simulink library "inp"  (Simulink 8.2) (continued)
== system inp/INP/InputSignals/SignalProcess/Proc_CameraSignals == (continued)
  [VINP_Obstacle6_BUS_InHAL] -> Bus Selector55/1
  [VINP_Obstacle7_BUS_InHAL] -> Bus Selector56/1
  [VINP_Obstacle8_BUS_InHAL] -> Bus Selector57/1
  [VINP_Obstacle9_BUS_InHAL] -> Bus Selector58/1
  [VINP_Obstacle10_BUS_InHAL] -> Bus Selector59/1
  Bus Selector59/1 -> Mux5/10  "<VINP_CamObs10PosYValid_flg_InHAL>"
  Mux5/1 -> VINP_CamObsPosYValid10_flg_InProc/1  "VINP_CamObsPosYValid10_flg_InProc"
  Bus Selector60/1 -> Mux6/1  "<VINP_CamObs1VelX_mps_InHAL>"
  Bus Selector61/1 -> Mux6/2  "<VINP_CamObs2VelX_mps_InHAL>"
  Bus Selector62/1 -> Mux6/3  "<VINP_CamObs3VelX_mps_InHAL>"
  Bus Selector63/1 -> Mux6/4  "<VINP_CamObs4VelX_mps_InHAL>"
  Bus Selector64/1 -> Mux6/5  "<VINP_CamObs5VelX_mps_InHAL>"
  Bus Selector65/1 -> Mux6/6  "<VINP_CamObs6VelX_mps_InHAL>"
  Bus Selector66/1 -> Mux6/7  "<VINP_CamObs7VelX_mps_InHAL>"
  Bus Selector67/1 -> Mux6/8  "<VINP_CamObs8VelX_mps_InHAL>"
  Bus Selector68/1 -> Mux6/9  "<VINP_CamObs9VelX_mps_InHAL>"
  [VINP_Obstacle1_BUS_InHAL] -> Bus Selector60/1
  [VINP_Obstacle2_BUS_InHAL] -> Bus Selector61/1
  [VINP_Obstacle3_BUS_InHAL] -> Bus Selector62/1
  [VINP_Obstacle4_BUS_InHAL] -> Bus Selector63/1
  [VINP_Obstacle5_BUS_InHAL] -> Bus Selector64/1
  [VINP_Obstacle6_BUS_InHAL] -> Bus Selector65/1
  [VINP_Obstacle7_BUS_InHAL] -> Bus Selector66/1
  [VINP_Obstacle8_BUS_InHAL] -> Bus Selector67/1
  [VINP_Obstacle9_BUS_InHAL] -> Bus Selector68/1
  [VINP_Obstacle10_BUS_InHAL] -> Bus Selector69/1
  Bus Selector69/1 -> Mux6/10  "<VINP_CamObs10VelX_mps_InHAL>"
  Mux6/1 -> VINP_CamObsVelX10_mps_InProc/1  "VINP_CamObsVelX10_mps_InProc"
  Bus Selector70/1 -> Mux7/1  "<VINP_CamObs1VelXValid_flg_InHAL>"
  Bus Selector71/1 -> Mux7/2  "<VINP_CamObs2VelXValid_flg_InHAL>"
  Bus Selector72/1 -> Mux7/3  "<VINP_CamObs3VelXValid_flg_InHAL>"
  Bus Selector73/1 -> Mux7/4  "<VINP_CamObs4VelXValid_flg_InHAL>"
  Bus Selector74/1 -> Mux7/5  "<VINP_CamObs5VelXValid_flg_InHAL>"
  Bus Selector75/1 -> Mux7/6  "<VINP_CamObs6VelXValid_flg_InHAL>"
  Bus Selector76/1 -> Mux7/7  "<VINP_CamObs7VelXValid_flg_InHAL>"
  Bus Selector77/1 -> Mux7/8  "<VINP_CamObs8VelXValid_flg_InHAL>"
  Bus Selector78/1 -> Mux7/9  "<VINP_CamObs9VelXValid_flg_InHAL>"
  [VINP_Obstacle1_BUS_InHAL] -> Bus Selector70/1
  [VINP_Obstacle2_BUS_InHAL] -> Bus Selector71/1
  [VINP_Obstacle3_BUS_InHAL] -> Bus Selector72/1
  [VINP_Obstacle4_BUS_InHAL] -> Bus Selector73/1
  [VINP_Obstacle5_BUS_InHAL] -> Bus Selector74/1
  [VINP_Obstacle6_BUS_InHAL] -> Bus Selector75/1
  [VINP_Obstacle7_BUS_InHAL] -> Bus Selector76/1
  [VINP_Obstacle8_BUS_InHAL] -> Bus Selector77/1
  [VINP_Obstacle9_BUS_InHAL] -> Bus Selector78/1
  [VINP_Obstacle10_BUS_InHAL] -> Bus Selector79/1
  Bus Selector79/1 -> Mux7/10  "<VINP_CamObs10VelXValid_flg_InHAL>"
  Mux7/1 -> VINP_CamObsVelXValid10_flg_InProc/1  "VINP_CamObsVelXValid10_flg_InProc"
  Bus Selector80/1 -> Mux8/1  "<VINP_CamObs1Type_enum_InHAL>"
  Bus Selector81/1 -> Mux8/2  "<VINP_CamObs2Type_enum_InHAL>"
  Bus Selector82/1 -> Mux8/3  "<VINP_CamObs3Type_enum_InHAL>"
  Bus Selector83/1 -> Mux8/4  "<VINP_CamObs4Type_enum_InHAL>"
  Bus Selector84/1 -> Mux8/5  "<VINP_CamObs5Type_enum_InHAL>"
  Bus Selector85/1 -> Mux8/6  "<VINP_CamObs6Type_enum_InHAL>"
  Bus Selector86/1 -> Mux8/7  "<VINP_CamObs7Type_enum_InHAL>"
  Bus Selector87/1 -> Mux8/8  "<VINP_CamObs8Type_enum_InHAL>"
  Bus Selector88/1 -> Mux8/9  "<VINP_CamObs9Type_enum_InHAL>"
  [VINP_Obstacle1_BUS_InHAL] -> Bus Selector80/1
  [VINP_Obstacle2_BUS_InHAL] -> Bus Selector81/1
  [VINP_Obstacle3_BUS_InHAL] -> Bus Selector82/1
  [VINP_Obstacle4_BUS_InHAL] -> Bus Selector83/1
  [VINP_Obstacle5_BUS_InHAL] -> Bus Selector84/1
  [VINP_Obstacle6_BUS_InHAL] -> Bus Selector85/1
  [VINP_Obstacle7_BUS_InHAL] -> Bus Selector86/1
  [VINP_Obstacle8_BUS_InHAL] -> Bus Selector87/1
  [VINP_Obstacle9_BUS_InHAL] -> Bus Selector88/1
  [VINP_Obstacle10_BUS_InHAL] -> Bus Selector89/1
  Bus Selector89/1 -> Mux8/10  "<VINP_CamObs10Type_enum_InHAL>"
  Mux8/1 -> VINP_CamObsType10_enum_InProc/1  "VINP_CamObsType10_enum_InProc"
  Bus Selector90/1 -> Mux9/1  "<VINP_CamObs1TypeValid_flg_InHAL>"
  Bus Selector91/1 -> Mux9/2  "<VINP_CamObs2TypeValid_flg_InHAL>"
  Bus Selector92/1 -> Mux9/3  "<VINP_CamObs3TypeValid_flg_InHAL>"
  Bus Selector93/1 -> Mux9/4  "<VINP_CamObs4TypeValid_flg_InHAL>"
  Bus Selector94/1 -> Mux9/5  "<VINP_CamObs5TypeValid_flg_InHAL>"
  Bus Selector95/1 -> Mux9/6  "<VINP_CamObs6TypeValid_flg_InHAL>"
  Bus Selector96/1 -> Mux9/7  "<VINP_CamObs7TypeValid_flg_InHAL>"
  Bus Selector97/1 -> Mux9/8  "<VINP_CamObs8TypeValid_flg_InHAL>"
  Bus Selector98/1 -> Mux9/9  "<VINP_CamObs9TypeValid_flg_InHAL>"
  [VINP_Obstacle1_BUS_InHAL] -> Bus Selector90/1
  [VINP_Obstacle2_BUS_InHAL] -> Bus Selector91/1
  [VINP_Obstacle3_BUS_InHAL] -> Bus Selector92/1
  [VINP_Obstacle4_BUS_InHAL] -> Bus Selector93/1
  [VINP_Obstacle5_BUS_InHAL] -> Bus Selector94/1
  [VINP_Obstacle6_BUS_InHAL] -> Bus Selector95/1
  [VINP_Obstacle7_BUS_InHAL] -> Bus Selector96/1
  [VINP_Obstacle8_BUS_InHAL] -> Bus Selector97/1
  [VINP_Obstacle9_BUS_InHAL] -> Bus Selector98/1
  [VINP_Obstacle10_BUS_InHAL] -> Bus Selector99/1
  Bus Selector99/1 -> Mux9/10  "<VINP_CamObs10TypeValid_flg_InHAL>"
  Mux9/1 -> VINP_CamObsTypeValid10_flg_InProc/1  "VINP_CamObsTypeValid10_flg_InProc"
  Bus Selector100/1 -> Mux10/1  "<VINP_CamObs1Status_enum_InHAL>"
  Bus Selector101/1 -> Mux10/2  "<VINP_CamObs2Status_enum_InHAL>"
  Bus Selector102/1 -> Mux10/3  "<VINP_CamObs3Status_enum_InHAL>"
  Bus Selector103/1 -> Mux10/4  "<VINP_CamObs4Status_enum_InHAL>"
  Bus Selector104/1 -> Mux10/5  "<VINP_CamObs5Status_enum_InHAL>"
  Bus Selector105/1 -> Mux10/6  "<VINP_CamObs6Status_enum_InHAL>"
  Bus Selector106/1 -> Mux10/7  "<VINP_CamObs7Status_enum_InHAL>"
  Bus Selector107/1 -> Mux10/8  "<VINP_CamObs8Status_enum_InHAL>"
  Bus Selector108/1 -> Mux10/9  "<VINP_CamObs9Status_enum_InHAL>"
  [VINP_Obstacle1_BUS_InHAL] -> Bus Selector100/1
  [VINP_Obstacle2_BUS_InHAL] -> Bus Selector101/1
  [VINP_Obstacle3_BUS_InHAL] -> Bus Selector102/1
  [VINP_Obstacle4_BUS_InHAL] -> Bus Selector103/1
  [VINP_Obstacle5_BUS_InHAL] -> Bus Selector104/1
  [VINP_Obstacle6_BUS_InHAL] -> Bus Selector105/1
  [VINP_Obstacle7_BUS_InHAL] -> Bus Selector106/1
  [VINP_Obstacle8_BUS_InHAL] -> Bus Selector107/1
  [VINP_Obstacle9_BUS_InHAL] -> Bus Selector108/1
  [VINP_Obstacle10_BUS_InHAL] -> Bus Selector109/1
  Bus Selector109/1 -> Mux10/10  "<VINP_CamObs10Status_enum_InHAL>"
  Mux10/1 -> VINP_CamObsStatus10_enum_InProc/1  "VINP_CamObsStatus10_enum_InProc"
  Bus Selector110/1 -> Mux11/1  "<VINP_CamObs1StatusValid_flg_InHAL>"
  Bus Selector111/1 -> Mux11/2  "<VINP_CamObs2StatusValid_flg_InHAL>"
  Bus Selector112/1 -> Mux11/3  "<VINP_CamObs3StatusValid_flg_InHAL>"
  Bus Selector113/1 -> Mux11/4  "<VINP_CamObs4StatusValid_flg_InHAL>"
  Bus Selector114/1 -> Mux11/5  "<VINP_CamObs5StatusValid_flg_InHAL>"
  Bus Selector115/1 -> Mux11/6  "<VINP_CamObs6StatusValid_flg_InHAL>"
  Bus Selector116/1 -> Mux11/7  "<VINP_CamObs7StatusValid_flg_InHAL>"
  Bus Selector117/1 -> Mux11/8  "<VINP_CamObs8StatusValid_flg_InHAL>"
  Bus Selector118/1 -> Mux11/9  "<VINP_CamObs9StatusValid_flg_InHAL>"
  [VINP_Obstacle1_BUS_InHAL] -> Bus Selector110/1
  [VINP_Obstacle2_BUS_InHAL] -> Bus Selector111/1
  [VINP_Obstacle3_BUS_InHAL] -> Bus Selector112/1
  [VINP_Obstacle4_BUS_InHAL] -> Bus Selector113/1
  [VINP_Obstacle5_BUS_InHAL] -> Bus Selector114/1
  [VINP_Obstacle6_BUS_InHAL] -> Bus Selector115/1
  [VINP_Obstacle7_BUS_InHAL] -> Bus Selector116/1
  [VINP_Obstacle8_BUS_InHAL] -> Bus Selector117/1
  [VINP_Obstacle9_BUS_InHAL] -> Bus Selector118/1
  [VINP_Obstacle10_BUS_InHAL] -> Bus Selector119/1
  Bus Selector119/1 -> Mux11/10  "<VINP_CamObs10StatusValid_flg_InHAL>"
  Mux11/1 -> VINP_CamObsStatusValid10_flg_InProc/1  "VINP_CamObsStatusValid10_flg_InProc"
  Bus Selector120/1 -> Mux12/1  "<VINP_CamObs1Valid_enum_InHAL>"
  Bus Selector121/1 -> Mux12/2  "<VINP_CamObs2Valid_enum_InHAL>"
  Bus Selector122/1 -> Mux12/3  "<VINP_CamObs3Valid_enum_InHAL>"
  Bus Selector123/1 -> Mux12/4  "<VINP_CamObs4Valid_enum_InHAL>"
  Bus Selector124/1 -> Mux12/5  "<VINP_CamObs5Valid_enum_InHAL>"
  Bus Selector125/1 -> Mux12/6  "<VINP_CamObs6Valid_enum_InHAL>"
  Bus Selector126/1 -> Mux12/7  "<VINP_CamObs7Valid_enum_InHAL>"
  Bus Selector127/1 -> Mux12/8  "<VINP_CamObs8Valid_enum_InHAL>"
  Bus Selector128/1 -> Mux12/9  "<VINP_CamObs9Valid_enum_InHAL>"
  [VINP_Obstacle1_BUS_InHAL] -> Bus Selector120/1
  [VINP_Obstacle2_BUS_InHAL] -> Bus Selector121/1
  [VINP_Obstacle3_BUS_InHAL] -> Bus Selector122/1
  [VINP_Obstacle4_BUS_InHAL] -> Bus Selector123/1
  [VINP_Obstacle5_BUS_InHAL] -> Bus Selector124/1
  [VINP_Obstacle6_BUS_InHAL] -> Bus Selector125/1
  [VINP_Obstacle7_BUS_InHAL] -> Bus Selector126/1
  [VINP_Obstacle8_BUS_InHAL] -> Bus Selector127/1
  [VINP_Obstacle9_BUS_InHAL] -> Bus Selector128/1
  [VINP_Obstacle10_BUS_InHAL] -> Bus Selector129/1
  Bus Selector129/1 -> Mux12/10  "<VINP_CamObs10Valid_enum_InHAL>"
  Mux12/1 -> VINP_CamObsValid10_enum_InProc/1  "VINP_CamObsValid10_enum_InProc"
  Bus Selector130/1 -> Mux13/1  "<VINP_CamObs1ValidValid_flg_InHAL>"
  Bus Selector131/1 -> Mux13/2  "<VINP_CamObs2ValidValid_flg_InHAL>"
  Bus Selector132/1 -> Mux13/3  "<VINP_CamObs3ValidValid_flg_InHAL>"
  Bus Selector133/1 -> Mux13/4  "<VINP_CamObs4ValidValid_flg_InHAL>"
  Bus Selector134/1 -> Mux13/5  "<VINP_CamObs5ValidValid_flg_InHAL>"
  Bus Selector135/1 -> Mux13/6  "<VINP_CamObs6ValidValid_flg_InHAL>"
  Bus Selector136/1 -> Mux13/7  "<VINP_CamObs7ValidValid_flg_InHAL>"
  Bus Selector137/1 -> Mux13/8  "<VINP_CamObs8ValidValid_flg_InHAL>"
  Bus Selector138/1 -> Mux13/9  "<VINP_CamObs9ValidValid_flg_InHAL>"
  [VINP_Obstacle1_BUS_InHAL] -> Bus Selector130/1
  [VINP_Obstacle2_BUS_InHAL] -> Bus Selector131/1
  [VINP_Obstacle3_BUS_InHAL] -> Bus Selector132/1
  [VINP_Obstacle4_BUS_InHAL] -> Bus Selector133/1
  [VINP_Obstacle5_BUS_InHAL] -> Bus Selector134/1
  [VINP_Obstacle6_BUS_InHAL] -> Bus Selector135/1
  [VINP_Obstacle7_BUS_InHAL] -> Bus Selector136/1
  [VINP_Obstacle8_BUS_InHAL] -> Bus Selector137/1
  [VINP_Obstacle9_BUS_InHAL] -> Bus Selector138/1
  [VINP_Obstacle10_BUS_InHAL] -> Bus Selector139/1
  Bus Selector139/1 -> Mux13/10  "<VINP_CamObs10ValidValid_flg_InHAL>"
  Mux13/1 -> VINP_CamObsValidValid10_flg_InProc/1  "VINP_CamObsValidValid10_flg_InProc"
  Bus Selector140/1 -> Mux14/1  "<VINP_CamObs1MoveInO_enum_InHAL>"
  Bus Selector141/1 -> Mux14/2  "<VINP_CamObs2MoveInO_enum_InHAL>"
  Bus Selector142/1 -> Mux14/3  "<VINP_CamObs3MoveInO_enum_InHAL>"
  Bus Selector143/1 -> Mux14/4  "<VINP_CamObs4MoveInO_enum_InHAL>"
  Bus Selector144/1 -> Mux14/5  "<VINP_CamObs5MoveInO_enum_InHAL>"
  Bus Selector145/1 -> Mux14/6  "<VINP_CamObs6MoveInO_enum_InHAL>"
  Bus Selector146/1 -> Mux14/7  "<VINP_CamObs7MoveInO_enum_InHAL>"
  Bus Selector147/1 -> Mux14/8  "<VINP_CamObs8MoveInO_enum_InHAL>"
  Bus Selector148/1 -> Mux14/9  "<VINP_CamObs9MoveInO_enum_InHAL>"
  [VINP_Obstacle1_BUS_InHAL] -> Bus Selector140/1
  [VINP_Obstacle2_BUS_InHAL] -> Bus Selector141/1
  [VINP_Obstacle3_BUS_InHAL] -> Bus Selector142/1
  [VINP_Obstacle4_BUS_InHAL] -> Bus Selector143/1
  [VINP_Obstacle5_BUS_InHAL] -> Bus Selector144/1
  [VINP_Obstacle6_BUS_InHAL] -> Bus Selector145/1
  [VINP_Obstacle7_BUS_InHAL] -> Bus Selector146/1
  [VINP_Obstacle8_BUS_InHAL] -> Bus Selector147/1
  [VINP_Obstacle9_BUS_InHAL] -> Bus Selector148/1
  Mux14/1 -> VINP_CamObsMoveInO10_enum_InProc/1  "VINP_CamObsMoveInO10_enum_InProc"
  [VINP_Obstacle10_BUS_InHAL] -> Bus Selector149/1
  Bus Selector149/1 -> Mux14/10  "<VINP_CamObs10MoveInO_enum_InHAL>"
  Bus Selector150/1 -> Mux15/1  "<VINP_CamObs1MoveInOValid_flg_InHAL>"
  Bus Selector151/1 -> Mux15/2  "<VINP_CamObs2MoveInOValid_flg_InHAL>"
  Bus Selector152/1 -> Mux15/3  "<VINP_CamObs3MoveInOValid_flg_InHAL>"
  Bus Selector153/1 -> Mux15/4  "<VINP_CamObs4MoveInOValid_flg_InHAL>"
  Bus Selector154/1 -> Mux15/5  "<VINP_CamObs5MoveInOValid_flg_InHAL>"
  Bus Selector155/1 -> Mux15/6  "<VINP_CamObs6MoveInOValid_flg_InHAL>"
  Bus Selector156/1 -> Mux15/7  "<VINP_CamObs7MoveInOValid_flg_InHAL>"
  Bus Selector157/1 -> Mux15/8  "<VINP_CamObs8MoveInOValid_flg_InHAL>"
  Bus Selector158/1 -> Mux15/9  "<VINP_CamObs9MoveInOValid_flg_InHAL>"
  [VINP_Obstacle1_BUS_InHAL] -> Bus Selector150/1
  [VINP_Obstacle2_BUS_InHAL] -> Bus Selector151/1
  [VINP_Obstacle3_BUS_InHAL] -> Bus Selector152/1
  [VINP_Obstacle4_BUS_InHAL] -> Bus Selector153/1
  [VINP_Obstacle5_BUS_InHAL] -> Bus Selector154/1
  [VINP_Obstacle6_BUS_InHAL] -> Bus Selector155/1
  [VINP_Obstacle7_BUS_InHAL] -> Bus Selector156/1
  [VINP_Obstacle8_BUS_InHAL] -> Bus Selector157/1
  [VINP_Obstacle9_BUS_InHAL] -> Bus Selector158/1
  Mux15/1 -> VINP_CamObsMoveInOValid10_flg_InProc/1  "VINP_CamObsMoveInOValid10_flg_InProc"
  [VINP_Obstacle10_BUS_InHAL] -> Bus Selector159/1
  Bus Selector159/1 -> Mux15/10  "<VINP_CamObs10MoveInOValid_flg_InHAL>"
  Bus Selector160/1 -> Mux16/1  "<VINP_CamObs1Blinker_enum_InHAL>"
  Bus Selector161/1 -> Mux16/2  "<VINP_CamObs2Blinker_enum_InHAL>"
  Bus Selector162/1 -> Mux16/3  "<VINP_CamObs3Blinker_enum_InHAL>"
  Bus Selector163/1 -> Mux16/4  "<VINP_CamObs4Blinker_enum_InHAL>"
  Bus Selector164/1 -> Mux16/5  "<VINP_CamObs5Blinker_enum_InHAL>"
  Bus Selector165/1 -> Mux16/6  "<VINP_CamObs6Blinker_enum_InHAL>"
  Bus Selector166/1 -> Mux16/7  "<VINP_CamObs7Blinker_enum_InHAL>"
  Bus Selector167/1 -> Mux16/8  "<VINP_CamObs8Blinker_enum_InHAL>"
  Bus Selector168/1 -> Mux16/9  "<VINP_CamObs9Blinker_enum_InHAL>"
  [VINP_Obstacle1_BUS_InHAL] -> Bus Selector160/1
  [VINP_Obstacle2_BUS_InHAL] -> Bus Selector161/1
  [VINP_Obstacle3_BUS_InHAL] -> Bus Selector162/1
  [VINP_Obstacle4_BUS_InHAL] -> Bus Selector163/1
  [VINP_Obstacle5_BUS_InHAL] -> Bus Selector164/1
  [VINP_Obstacle6_BUS_InHAL] -> Bus Selector165/1
  [VINP_Obstacle7_BUS_InHAL] -> Bus Selector166/1
  [VINP_Obstacle8_BUS_InHAL] -> Bus Selector167/1
  [VINP_Obstacle9_BUS_InHAL] -> Bus Selector168/1
  Mux16/1 -> VINP_CamObsBlinker10_enum_InProc/1  "VINP_CamObsBlinker10_enum_InProc"
  [VINP_Obstacle10_BUS_InHAL] -> Bus Selector169/1
  Bus Selector169/1 -> Mux16/10  "<VINP_CamObs10Blinker_enum_InHAL>"
  Bus Selector170/1 -> Mux17/1  "<VINP_CamObs1BlinkerValid_flg_InHAL>"
  Bus Selector171/1 -> Mux17/2  "<VINP_CamObs2BlinkerValid_flg_InHAL>"
  Bus Selector172/1 -> Mux17/3  "<VINP_CamObs3BlinkerValid_flg_InHAL>"
  Bus Selector173/1 -> Mux17/4  "<VINP_CamObs4BlinkerValid_flg_InHAL>"
  Bus Selector174/1 -> Mux17/5  "<VINP_CamObs5BlinkerValid_flg_InHAL>"
  Bus Selector175/1 -> Mux17/6  "<VINP_CamObs6BlinkerValid_flg_InHAL>"
  Bus Selector176/1 -> Mux17/7  "<VINP_CamObs7BlinkerValid_flg_InHAL>"
  Bus Selector177/1 -> Mux17/8  "<VINP_CamObs8BlinkerValid_flg_InHAL>"
  Bus Selector178/1 -> Mux17/9  "<VINP_CamObs9BlinkerValid_flg_InHAL>"
  [VINP_Obstacle1_BUS_InHAL] -> Bus Selector170/1
  [VINP_Obstacle2_BUS_InHAL] -> Bus Selector171/1
  [VINP_Obstacle3_BUS_InHAL] -> Bus Selector172/1
  [VINP_Obstacle4_BUS_InHAL] -> Bus Selector173/1
  [VINP_Obstacle5_BUS_InHAL] -> Bus Selector174/1
  [VINP_Obstacle6_BUS_InHAL] -> Bus Selector175/1
  [VINP_Obstacle7_BUS_InHAL] -> Bus Selector176/1
  [VINP_Obstacle8_BUS_InHAL] -> Bus Selector177/1
  [VINP_Obstacle9_BUS_InHAL] -> Bus Selector178/1
  Mux17/1 -> VINP_CamObsBlinkerValid10_flg_InProc/1  "VINP_CamObsBlinkerValid10_flg_InProc"
  [VINP_Obstacle10_BUS_InHAL] -> Bus Selector179/1
  Bus Selector179/1 -> Mux17/10  "<VINP_CamObs10BlinkerValid_flg_InHAL>"
  Bus Selector180/1 -> Mux18/1  "<VINP_CamObs1BrakeLight_flg_InHAL>"
  Bus Selector181/1 -> Mux18/2  "<VINP_CamObs2BrakeLight_flg_InHAL>"
  Bus Selector182/1 -> Mux18/3  "<VINP_CamObs3BrakeLight_flg_InHAL>"
  Bus Selector183/1 -> Mux18/4  "<VINP_CamObs4BrakeLight_flg_InHAL>"
  Bus Selector184/1 -> Mux18/5  "<VINP_CamObs5BrakeLight_flg_InHAL>"
  Bus Selector185/1 -> Mux18/6  "<VINP_CamObs6BrakeLight_flg_InHAL>"
  Bus Selector186/1 -> Mux18/7  "<VINP_CamObs7BrakeLight_flg_InHAL>"
  Bus Selector187/1 -> Mux18/8  "<VINP_CamObs8BrakeLight_flg_InHAL>"
  Bus Selector188/1 -> Mux18/9  "<VINP_CamObs9BrakeLight_flg_InHAL>"
  [VINP_Obstacle1_BUS_InHAL] -> Bus Selector180/1
  [VINP_Obstacle2_BUS_InHAL] -> Bus Selector181/1
  [VINP_Obstacle3_BUS_InHAL] -> Bus Selector182/1
  [VINP_Obstacle4_BUS_InHAL] -> Bus Selector183/1
  [VINP_Obstacle5_BUS_InHAL] -> Bus Selector184/1
  [VINP_Obstacle6_BUS_InHAL] -> Bus Selector185/1
  [VINP_Obstacle7_BUS_InHAL] -> Bus Selector186/1
  [VINP_Obstacle8_BUS_InHAL] -> Bus Selector187/1
  [VINP_Obstacle9_BUS_InHAL] -> Bus Selector188/1
  Mux18/1 -> VINP_CamObsBrakeLight10_flg_InProc/1  "VINP_CamObsBrakeLight10_flg_InProc"
  [VINP_Obstacle10_BUS_InHAL] -> Bus Selector189/1
  Bus Selector189/1 -> Mux18/10  "<VINP_CamObs10BrakeLight_flg_InHAL>"
  Bus Selector190/1 -> Mux19/1  "<VINP_CamObs1BrakeLightValid_flg_InHAL>"
  Bus Selector191/1 -> Mux19/2  "<VINP_CamObs2BrakeLightValid_flg_InHAL>"
  Bus Selector192/1 -> Mux19/3  "<VINP_CamObs3BrakeLightValid_flg_InHAL>"
  Bus Selector193/1 -> Mux19/4  "<VINP_CamObs4BrakeLightValid_flg_InHAL>"
  Bus Selector194/1 -> Mux19/5  "<VINP_CamObs5BrakeLightValid_flg_InHAL>"
  Bus Selector195/1 -> Mux19/6  "<VINP_CamObs6BrakeLightValid_flg_InHAL>"
  Bus Selector196/1 -> Mux19/7  "<VINP_CamObs7BrakeLightValid_flg_InHAL>"
  Bus Selector197/1 -> Mux19/8  "<VINP_CamObs8BrakeLightValid_flg_InHAL>"
  Bus Selector198/1 -> Mux19/9  "<VINP_CamObs9BrakeLightValid_flg_InHAL>"
  [VINP_Obstacle1_BUS_InHAL] -> Bus Selector190/1
  [VINP_Obstacle2_BUS_InHAL] -> Bus Selector191/1
  [VINP_Obstacle3_BUS_InHAL] -> Bus Selector192/1
  [VINP_Obstacle4_BUS_InHAL] -> Bus Selector193/1
  [VINP_Obstacle5_BUS_InHAL] -> Bus Selector194/1
  [VINP_Obstacle6_BUS_InHAL] -> Bus Selector195/1
  [VINP_Obstacle7_BUS_InHAL] -> Bus Selector196/1
  [VINP_Obstacle8_BUS_InHAL] -> Bus Selector197/1
  [VINP_Obstacle9_BUS_InHAL] -> Bus Selector198/1
  Mux19/1 -> VINP_CamObsBrakeLightValid10_flg_InProc/1  "VINP_CamObsBrakeLightValid10_flg_InProc"
  [VINP_Obstacle10_BUS_InHAL] -> Bus Selector199/1
  Bus Selector199/1 -> Mux19/10  "<VINP_CamObs10BrakeLightValid_flg_InHAL>"
  Bus Selector200/1 -> Mux20/1  "<VINP_CamObs1Length_m_InHAL>"
  Bus Selector211/1 -> Mux20/2  "<VINP_CamObs2Length_m_InHAL>"
  Bus Selector222/1 -> Mux20/3  "<VINP_CamObs3Length_m_InHAL>"
  Bus Selector233/1 -> Mux20/4  "<VINP_CamObs4Length_m_InHAL>"
  Bus Selector244/1 -> Mux20/5  "<VINP_CamObs5Length_m_InHAL>"
  Bus Selector255/1 -> Mux20/6  "<VINP_CamObs6Length_m_InHAL>"
  Bus Selector266/1 -> Mux20/7  "<VINP_CamObs7Length_m_InHAL>"
  Bus Selector277/1 -> Mux20/8  "<VINP_CamObs8Length_m_InHAL>"
  Bus Selector288/1 -> Mux20/9  "<VINP_CamObs9Length_m_InHAL>"
  [VINP_Obstacle1_BUS_InHAL] -> Bus Selector200/1
  [VINP_Obstacle2_BUS_InHAL] -> Bus Selector211/1
  [VINP_Obstacle3_BUS_InHAL] -> Bus Selector222/1
  [VINP_Obstacle4_BUS_InHAL] -> Bus Selector233/1
  [VINP_Obstacle5_BUS_InHAL] -> Bus Selector244/1
  [VINP_Obstacle6_BUS_InHAL] -> Bus Selector255/1
  [VINP_Obstacle7_BUS_InHAL] -> Bus Selector266/1
  [VINP_Obstacle8_BUS_InHAL] -> Bus Selector277/1
  [VINP_Obstacle9_BUS_InHAL] -> Bus Selector288/1
  [VINP_Obstacle10_BUS_InHAL] -> Bus Selector299/1
  Bus Selector299/1 -> Mux20/10  "<VINP_CamObs10Length_m_InHAL>"
  Mux20/1 -> VINP_CamObsLength10_m_InProc/1  "VINP_CamObsLength10_m_InProc"
  Bus Selector310/1 -> Mux31/1  "<VINP_CamObs1LengthValid_flg_InHAL>"
  Bus Selector311/1 -> Mux31/2  "<VINP_CamObs2LengthValid_flg_InHAL>"
  Bus Selector312/1 -> Mux31/3  "<VINP_CamObs3LengthValid_flg_InHAL>"
  Bus Selector313/1 -> Mux31/4  "<VINP_CamObs4LengthValid_flg_InHAL>"
  Bus Selector314/1 -> Mux31/5  "<VINP_CamObs5LengthValid_flg_InHAL>"
  Bus Selector315/1 -> Mux31/6  "<VINP_CamObs6LengthValid_flg_InHAL>"
  Bus Selector316/1 -> Mux31/7  "<VINP_CamObs7LengthValid_flg_InHAL>"
  Bus Selector317/1 -> Mux31/8  "<VINP_CamObs8LengthValid_flg_InHAL>"
  Bus Selector318/1 -> Mux31/9  "<VINP_CamObs9LengthValid_flg_InHAL>"
  [VINP_Obstacle1_BUS_InHAL] -> Bus Selector310/1
  [VINP_Obstacle2_BUS_InHAL] -> Bus Selector311/1
  [VINP_Obstacle3_BUS_InHAL] -> Bus Selector312/1
  [VINP_Obstacle4_BUS_InHAL] -> Bus Selector313/1
  [VINP_Obstacle5_BUS_InHAL] -> Bus Selector314/1
  [VINP_Obstacle6_BUS_InHAL] -> Bus Selector315/1
  [VINP_Obstacle7_BUS_InHAL] -> Bus Selector316/1
  [VINP_Obstacle8_BUS_InHAL] -> Bus Selector317/1
  [VINP_Obstacle9_BUS_InHAL] -> Bus Selector318/1
  [VINP_Obstacle10_BUS_InHAL] -> Bus Selector319/1
  Bus Selector319/1 -> Mux31/10  "<VINP_CamObs10LengthValid_flg_InHAL>"
  Mux31/1 -> VINP_CamObsLengthValid10_flg_InProc/1  "VINP_CamObsLengthValid10_flg_InProc"
  Bus Selector320/1 -> Mux32/1  "<VINP_CamObs1Width_m_InHAL>"
  Bus Selector321/1 -> Mux32/2  "<VINP_CamObs2Width_m_InHAL>"
  Bus Selector322/1 -> Mux32/3  "<VINP_CamObs3Width_m_InHAL>"
  Bus Selector323/1 -> Mux32/4  "<VINP_CamObs4Width_m_InHAL>"
  Bus Selector324/1 -> Mux32/5  "<VINP_CamObs5Width_m_InHAL>"
  Bus Selector325/1 -> Mux32/6  "<VINP_CamObs6Width_m_InHAL>"
  Bus Selector326/1 -> Mux32/7  "<VINP_CamObs7Width_m_InHAL>"
  Bus Selector327/1 -> Mux32/8  "<VINP_CamObs8Width_m_InHAL>"
  Bus Selector328/1 -> Mux32/9  "<VINP_CamObs9Width_m_InHAL>"
  [VINP_Obstacle1_BUS_InHAL] -> Bus Selector320/1
  [VINP_Obstacle2_BUS_InHAL] -> Bus Selector321/1
  [VINP_Obstacle3_BUS_InHAL] -> Bus Selector322/1
  [VINP_Obstacle4_BUS_InHAL] -> Bus Selector323/1
  [VINP_Obstacle5_BUS_InHAL] -> Bus Selector324/1
  [VINP_Obstacle6_BUS_InHAL] -> Bus Selector325/1
  [VINP_Obstacle7_BUS_InHAL] -> Bus Selector326/1
  [VINP_Obstacle8_BUS_InHAL] -> Bus Selector327/1
  [VINP_Obstacle9_BUS_InHAL] -> Bus Selector328/1
  [VINP_Obstacle10_BUS_InHAL] -> Bus Selector329/1
  Bus Selector329/1 -> Mux32/10  "<VINP_CamObs10Width_m_InHAL>"
  Mux32/1 -> VINP_CamObsWidth10_m_InProc/1  "VINP_CamObsWidth10_m_InProc"
  Bus Selector330/1 -> Mux33/1  "<VINP_CamObs1WidthValid_flg_InHAL>"
  Bus Selector331/1 -> Mux33/2  "<VINP_CamObs2WidthValid_flg_InHAL>"
  Bus Selector332/1 -> Mux33/3  "<VINP_CamObs3WidthValid_flg_InHAL>"
  Bus Selector333/1 -> Mux33/4  "<VINP_CamObs4WidthValid_flg_InHAL>"
  Bus Selector334/1 -> Mux33/5  "<VINP_CamObs5WidthValid_flg_InHAL>"
  Bus Selector335/1 -> Mux33/6  "<VINP_CamObs6WidthValid_flg_InHAL>"
  Bus Selector336/1 -> Mux33/7  "<VINP_CamObs7WidthValid_flg_InHAL>"
  Bus Selector337/1 -> Mux33/8  "<VINP_CamObs8WidthValid_flg_InHAL>"
  Bus Selector338/1 -> Mux33/9  "<VINP_CamObs9WidthValid_flg_InHAL>"
  [VINP_Obstacle1_BUS_InHAL] -> Bus Selector330/1
  [VINP_Obstacle2_BUS_InHAL] -> Bus Selector331/1
  [VINP_Obstacle3_BUS_InHAL] -> Bus Selector332/1
  [VINP_Obstacle4_BUS_InHAL] -> Bus Selector333/1
  [VINP_Obstacle5_BUS_InHAL] -> Bus Selector334/1
  [VINP_Obstacle6_BUS_InHAL] -> Bus Selector335/1
  [VINP_Obstacle7_BUS_InHAL] -> Bus Selector336/1
  [VINP_Obstacle8_BUS_InHAL] -> Bus Selector337/1
  [VINP_Obstacle9_BUS_InHAL] -> Bus Selector338/1
  [VINP_Obstacle10_BUS_InHAL] -> Bus Selector339/1
  Bus Selector339/1 -> Mux33/10  "<VINP_CamObs10WidthValid_flg_InHAL>"
  Mux33/1 -> VINP_CamObsWidthValid10_flg_InProc/1  "VINP_CamObsWidthValid10_flg_InProc"
  Bus Selector340/1 -> Mux34/1  "<VINP_CamObs1Age_cnt_InHAL>"
  Bus Selector341/1 -> Mux34/2  "<VINP_CamObs2Age_cnt_InHAL>"
  Bus Selector342/1 -> Mux34/3  "<VINP_CamObs3Age_cnt_InHAL>"
  Bus Selector343/1 -> Mux34/4  "<VINP_CamObs4Age_cnt_InHAL>"
  Bus Selector344/1 -> Mux34/5  "<VINP_CamObs5Age_cnt_InHAL>"
  Bus Selector345/1 -> Mux34/6  "<VINP_CamObs6Age_cnt_InHAL>"
  Bus Selector346/1 -> Mux34/7  "<VINP_CamObs7Age_cnt_InHAL>"
  Bus Selector347/1 -> Mux34/8  "<VINP_CamObs8Age_cnt_InHAL>"
  Bus Selector348/1 -> Mux34/9  "<VINP_CamObs9Age_cnt_InHAL>"
  [VINP_Obstacle1_BUS_InHAL] -> Bus Selector340/1
  [VINP_Obstacle2_BUS_InHAL] -> Bus Selector341/1
  [VINP_Obstacle3_BUS_InHAL] -> Bus Selector342/1
  [VINP_Obstacle4_BUS_InHAL] -> Bus Selector343/1
  [VINP_Obstacle5_BUS_InHAL] -> Bus Selector344/1
  [VINP_Obstacle6_BUS_InHAL] -> Bus Selector345/1
  [VINP_Obstacle7_BUS_InHAL] -> Bus Selector346/1
  [VINP_Obstacle8_BUS_InHAL] -> Bus Selector347/1
  [VINP_Obstacle9_BUS_InHAL] -> Bus Selector348/1
  [VINP_Obstacle10_BUS_InHAL] -> Bus Selector349/1
  Bus Selector349/1 -> Mux34/10  "<VINP_CamObs10Age_cnt_InHAL>"
  Mux34/1 -> VINP_CamObsAge10_cnt_InProc/1  "VINP_CamObsAge10_cnt_InProc"
  Bus Selector350/1 -> Mux35/1  "<VINP_CamObs1AgeValid_flg_InHAL>"
  Bus Selector351/1 -> Mux35/2  "<VINP_CamObs2AgeValid_flg_InHAL>"
  Bus Selector352/1 -> Mux35/3  "<VINP_CamObs3AgeValid_flg_InHAL>"
  Bus Selector353/1 -> Mux35/4  "<VINP_CamObs4AgeValid_flg_InHAL>"
  Bus Selector354/1 -> Mux35/5  "<VINP_CamObs5AgeValid_flg_InHAL>"
  Bus Selector355/1 -> Mux35/6  "<VINP_CamObs6AgeValid_flg_InHAL>"
  Bus Selector356/1 -> Mux35/7  "<VINP_CamObs7AgeValid_flg_InHAL>"
  Bus Selector357/1 -> Mux35/8  "<VINP_CamObs8AgeValid_flg_InHAL>"
  Bus Selector358/1 -> Mux35/9  "<VINP_CamObs9AgeValid_flg_InHAL>"
  [VINP_Obstacle1_BUS_InHAL] -> Bus Selector350/1
  [VINP_Obstacle2_BUS_InHAL] -> Bus Selector351/1
  [VINP_Obstacle3_BUS_InHAL] -> Bus Selector352/1
  [VINP_Obstacle4_BUS_InHAL] -> Bus Selector353/1
  [VINP_Obstacle5_BUS_InHAL] -> Bus Selector354/1
  [VINP_Obstacle6_BUS_InHAL] -> Bus Selector355/1
  [VINP_Obstacle7_BUS_InHAL] -> Bus Selector356/1
  [VINP_Obstacle8_BUS_InHAL] -> Bus Selector357/1
  [VINP_Obstacle9_BUS_InHAL] -> Bus Selector358/1
  [VINP_Obstacle10_BUS_InHAL] -> Bus Selector359/1
  Bus Selector359/1 -> Mux35/10  "<VINP_CamObs10AgeValid_flg_InHAL>"
  Mux35/1 -> VINP_CamObsAgeValid10_flg_InProc/1  "VINP_CamObsAgeValid10_flg_InProc"
  Bus Selector360/1 -> Mux36/1  "<VINP_CamObs1Lane_enum_InHAL>"
  Bus Selector361/1 -> Mux36/2  "<VINP_CamObs2Lane_enum_InHAL>"
  Bus Selector362/1 -> Mux36/3  "<VINP_CamObs3Lane_enum_InHAL>"
  Bus Selector363/1 -> Mux36/4  "<VINP_CamObs4Lane_enum_InHAL>"
  Bus Selector364/1 -> Mux36/5  "<VINP_CamObs5Lane_enum_InHAL>"
  Bus Selector365/1 -> Mux36/6  "<VINP_CamObs6Lane_enum_InHAL>"
  Bus Selector366/1 -> Mux36/7  "<VINP_CamObs7Lane_enum_InHAL>"
  Bus Selector367/1 -> Mux36/8  "<VINP_CamObs8Lane_enum_InHAL>"
  Bus Selector368/1 -> Mux36/9  "<VINP_CamObs9Lane_enum_InHAL>"
  [VINP_Obstacle1_BUS_InHAL] -> Bus Selector360/1
  [VINP_Obstacle2_BUS_InHAL] -> Bus Selector361/1
  [VINP_Obstacle3_BUS_InHAL] -> Bus Selector362/1
  [VINP_Obstacle4_BUS_InHAL] -> Bus Selector363/1
  [VINP_Obstacle5_BUS_InHAL] -> Bus Selector364/1
  [VINP_Obstacle6_BUS_InHAL] -> Bus Selector365/1
  [VINP_Obstacle7_BUS_InHAL] -> Bus Selector366/1
  [VINP_Obstacle8_BUS_InHAL] -> Bus Selector367/1
  [VINP_Obstacle9_BUS_InHAL] -> Bus Selector368/1
  [VINP_Obstacle10_BUS_InHAL] -> Bus Selector369/1
  Bus Selector369/1 -> Mux36/10  "<VINP_CamObs10Lane_enum_InHAL>"
  Mux36/1 -> VINP_CamObsLane10_enum_InProc/1  "VINP_CamObsLane10_enum_InProc"
  Bus Selector370/1 -> Mux37/1  "<VINP_CamObs1LaneValid_flg_InHAL>"
  Bus Selector371/1 -> Mux37/2  "<VINP_CamObs2LaneValid_flg_InHAL>"
  Bus Selector372/1 -> Mux37/3  "<VINP_CamObs3LaneValid_flg_InHAL>"
  Bus Selector373/1 -> Mux37/4  "<VINP_CamObs4LaneValid_flg_InHAL>"
  Bus Selector374/1 -> Mux37/5  "<VINP_CamObs5LaneValid_flg_InHAL>"
  Bus Selector375/1 -> Mux37/6  "<VINP_CamObs6LaneValid_flg_InHAL>"
  Bus Selector376/1 -> Mux37/7  "<VINP_CamObs7LaneValid_flg_InHAL>"
  Bus Selector377/1 -> Mux37/8  "<VINP_CamObs8LaneValid_flg_InHAL>"
  Bus Selector378/1 -> Mux37/9  "<VINP_CamObs9LaneValid_flg_InHAL>"
  [VINP_Obstacle1_BUS_InHAL] -> Bus Selector370/1
  [VINP_Obstacle2_BUS_InHAL] -> Bus Selector371/1
  [VINP_Obstacle3_BUS_InHAL] -> Bus Selector372/1
  [VINP_Obstacle4_BUS_InHAL] -> Bus Selector373/1
  [VINP_Obstacle5_BUS_InHAL] -> Bus Selector374/1
  [VINP_Obstacle6_BUS_InHAL] -> Bus Selector375/1
  [VINP_Obstacle7_BUS_InHAL] -> Bus Selector376/1
  [VINP_Obstacle8_BUS_InHAL] -> Bus Selector377/1
  [VINP_Obstacle9_BUS_InHAL] -> Bus Selector378/1
  [VINP_Obstacle10_BUS_InHAL] -> Bus Selector379/1
  Bus Selector379/1 -> Mux37/10  "<VINP_CamObs10LaneValid_flg_InHAL>"
  Mux37/1 -> VINP_CamObsLaneValid10_flg_InProc/1  "VINP_CamObsLaneValid10_flg_InProc"
  Bus Selector380/1 -> Mux38/1  "<VINP_CamObs1CIPV_flg_InHAL>"
  Bus Selector381/1 -> Mux38/2  "<VINP_CamObs2CIPV_flg_InHAL>"
  Bus Selector382/1 -> Mux38/3  "<VINP_CamObs3CIPV_flg_InHAL>"
  Bus Selector383/1 -> Mux38/4  "<VINP_CamObs4CIPV_flg_InHAL>"
  Bus Selector384/1 -> Mux38/5  "<VINP_CamObs5CIPV_flg_InHAL>"
  Bus Selector385/1 -> Mux38/6  "<VINP_CamObs6CIPV_flg_InHAL>"
  Bus Selector386/1 -> Mux38/7  "<VINP_CamObs7CIPV_flg_InHAL>"
  Bus Selector387/1 -> Mux38/8  "<VINP_CamObs8CIPV_flg_InHAL>"
  Bus Selector388/1 -> Mux38/9  "<VINP_CamObs9CIPV_flg_InHAL>"
  [VINP_Obstacle1_BUS_InHAL] -> Bus Selector380/1
  [VINP_Obstacle2_BUS_InHAL] -> Bus Selector381/1
  [VINP_Obstacle3_BUS_InHAL] -> Bus Selector382/1
  [VINP_Obstacle4_BUS_InHAL] -> Bus Selector383/1
  [VINP_Obstacle5_BUS_InHAL] -> Bus Selector384/1
  [VINP_Obstacle6_BUS_InHAL] -> Bus Selector385/1
  [VINP_Obstacle7_BUS_InHAL] -> Bus Selector386/1
  [VINP_Obstacle8_BUS_InHAL] -> Bus Selector387/1
  [VINP_Obstacle9_BUS_InHAL] -> Bus Selector388/1
  [VINP_Obstacle10_BUS_InHAL] -> Bus Selector389/1
  Bus Selector389/1 -> Mux38/10  "<VINP_CamObs10CIPV_flg_InHAL>"
  Mux38/1 -> VINP_CamObsCIPV10_flg_InProc/1  "VINP_CamObsCIPV10_flg_InProc"
  Bus Selector201/1 -> Mux21/1  "<VINP_CamObs1CIPVValid_flg_InHAL>"
  Bus Selector202/1 -> Mux21/2  "<VINP_CamObs2CIPVValid_flg_InHAL>"
  Bus Selector203/1 -> Mux21/3  "<VINP_CamObs3CIPVValid_flg_InHAL>"
  Bus Selector204/1 -> Mux21/4  "<VINP_CamObs4CIPVValid_flg_InHAL>"
  Bus Selector205/1 -> Mux21/5  "<VINP_CamObs5CIPVValid_flg_InHAL>"
  Bus Selector206/1 -> Mux21/6  "<VINP_CamObs6CIPVValid_flg_InHAL>"
  Bus Selector207/1 -> Mux21/7  "<VINP_CamObs7CIPVValid_flg_InHAL>"
  Bus Selector208/1 -> Mux21/8  "<VINP_CamObs8CIPVValid_flg_InHAL>"
  Bus Selector209/1 -> Mux21/9  "<VINP_CamObs9CIPVValid_flg_InHAL>"
  [VINP_Obstacle1_BUS_InHAL] -> Bus Selector201/1
  [VINP_Obstacle2_BUS_InHAL] -> Bus Selector202/1
  [VINP_Obstacle3_BUS_InHAL] -> Bus Selector203/1
  [VINP_Obstacle4_BUS_InHAL] -> Bus Selector204/1
  [VINP_Obstacle5_BUS_InHAL] -> Bus Selector205/1
  [VINP_Obstacle6_BUS_InHAL] -> Bus Selector206/1
  [VINP_Obstacle7_BUS_InHAL] -> Bus Selector207/1
  [VINP_Obstacle8_BUS_InHAL] -> Bus Selector208/1
  [VINP_Obstacle9_BUS_InHAL] -> Bus Selector209/1
  [VINP_Obstacle10_BUS_InHAL] -> Bus Selector210/1
  Bus Selector210/1 -> Mux21/10  "<VINP_CamObs10CIPVValid_flg_InHAL>"
  Mux21/1 -> VINP_CamObsCIPVValid10_flg_InProc/1  "VINP_CamObsCIPVValid10_flg_InProc"
  Bus Selector212/1 -> Mux22/1  "<VINP_CamObs1VelY_mps_InHAL>"
  Bus Selector213/1 -> Mux22/2  "<VINP_CamObs2VelY_mps_InHAL>"
  Bus Selector214/1 -> Mux22/3  "<VINP_CamObs3VelY_mps_InHAL>"
  Bus Selector215/1 -> Mux22/4  "<VINP_CamObs4VelY_mps_InHAL>"
  Bus Selector216/1 -> Mux22/5  "<VINP_CamObs5VelY_mps_InHAL>"
  Bus Selector217/1 -> Mux22/6  "<VINP_CamObs6VelY_mps_InHAL>"
  Bus Selector218/1 -> Mux22/7  "<VINP_CamObs7VelY_mps_InHAL>"
  Bus Selector219/1 -> Mux22/8  "<VINP_CamObs8VelY_mps_InHAL>"
  Bus Selector220/1 -> Mux22/9  "<VINP_CamObs9VelY_mps_InHAL>"
  [VINP_Obstacle1_BUS_InHAL] -> Bus Selector212/1
  [VINP_Obstacle2_BUS_InHAL] -> Bus Selector213/1
  [VINP_Obstacle3_BUS_InHAL] -> Bus Selector214/1
  [VINP_Obstacle4_BUS_InHAL] -> Bus Selector215/1
  [VINP_Obstacle5_BUS_InHAL] -> Bus Selector216/1
  [VINP_Obstacle6_BUS_InHAL] -> Bus Selector217/1
  [VINP_Obstacle7_BUS_InHAL] -> Bus Selector218/1
  [VINP_Obstacle8_BUS_InHAL] -> Bus Selector219/1
  [VINP_Obstacle9_BUS_InHAL] -> Bus Selector220/1
  [VINP_Obstacle10_BUS_InHAL] -> Bus Selector221/1
  Bus Selector221/1 -> Mux22/10  "<VINP_CamObs10VelY_mps_InHAL>"
  Mux22/1 -> VINP_CamObsVelY10_mps_InProc/1  "VINP_CamObsVelY10_mps_InProc"
  Bus Selector223/1 -> Mux23/1  "<VINP_CamObs1VelYValid_flg_InHAL>"
  Bus Selector224/1 -> Mux23/2  "<VINP_CamObs2VelYValid_flg_InHAL>"
  Bus Selector225/1 -> Mux23/3  "<VINP_CamObs3VelYValid_flg_InHAL>"
  Bus Selector226/1 -> Mux23/4  "<VINP_CamObs4VelYValid_flg_InHAL>"
  Bus Selector227/1 -> Mux23/5  "<VINP_CamObs5VelYValid_flg_InHAL>"
  Bus Selector228/1 -> Mux23/6  "<VINP_CamObs6VelYValid_flg_InHAL>"
  Bus Selector229/1 -> Mux23/7  "<VINP_CamObs7VelYValid_flg_InHAL>"
  Bus Selector230/1 -> Mux23/8  "<VINP_CamObs8VelYValid_flg_InHAL>"
  Bus Selector231/1 -> Mux23/9  "<VINP_CamObs9VelYValid_flg_InHAL>"
  [VINP_Obstacle1_BUS_InHAL] -> Bus Selector223/1
  [VINP_Obstacle2_BUS_InHAL] -> Bus Selector224/1
  [VINP_Obstacle3_BUS_InHAL] -> Bus Selector225/1
  [VINP_Obstacle4_BUS_InHAL] -> Bus Selector226/1
  [VINP_Obstacle5_BUS_InHAL] -> Bus Selector227/1
  [VINP_Obstacle6_BUS_InHAL] -> Bus Selector228/1
  [VINP_Obstacle7_BUS_InHAL] -> Bus Selector229/1
  [VINP_Obstacle8_BUS_InHAL] -> Bus Selector230/1
  [VINP_Obstacle9_BUS_InHAL] -> Bus Selector231/1
  [VINP_Obstacle10_BUS_InHAL] -> Bus Selector232/1
  Bus Selector232/1 -> Mux23/10  "<VINP_CamObs10VelYValid_flg_InHAL>"
  Mux23/1 -> VINP_CamObsVelYValid10_flg_InProc/1  "VINP_CamObsVelYValid10_flg_InProc"
  Bus Selector234/1 -> Mux24/1  "<VINP_CamObs1AccX_mps2_InHAL>"
  Bus Selector235/1 -> Mux24/2  "<VINP_CamObs2AccX_mps2_InHAL>"
  Bus Selector236/1 -> Mux24/3  "<VINP_CamObs3AccX_mps2_InHAL>"
  Bus Selector237/1 -> Mux24/4  "<VINP_CamObs4AccX_mps2_InHAL>"
  Bus Selector238/1 -> Mux24/5  "<VINP_CamObs5AccX_mps2_InHAL>"
  Bus Selector239/1 -> Mux24/6  "<VINP_CamObs6AccX_mps2_InHAL>"
  Bus Selector240/1 -> Mux24/7  "<VINP_CamObs7AccX_mps2_InHAL>"
  Bus Selector241/1 -> Mux24/8  "<VINP_CamObs8AccX_mps2_InHAL>"
  Bus Selector242/1 -> Mux24/9  "<VINP_CamObs9AccX_mps2_InHAL>"
  [VINP_Obstacle1_BUS_InHAL] -> Bus Selector234/1
  [VINP_Obstacle2_BUS_InHAL] -> Bus Selector235/1
  [VINP_Obstacle3_BUS_InHAL] -> Bus Selector236/1
  [VINP_Obstacle4_BUS_InHAL] -> Bus Selector237/1
  [VINP_Obstacle5_BUS_InHAL] -> Bus Selector238/1
  [VINP_Obstacle6_BUS_InHAL] -> Bus Selector239/1
  [VINP_Obstacle7_BUS_InHAL] -> Bus Selector240/1
  [VINP_Obstacle8_BUS_InHAL] -> Bus Selector241/1
  [VINP_Obstacle9_BUS_InHAL] -> Bus Selector242/1
  [VINP_Obstacle10_BUS_InHAL] -> Bus Selector243/1
  Bus Selector243/1 -> Mux24/10  "<VINP_CamObs10AccX_mps2_InHAL>"
  Mux24/1 -> VINP_CamObsAccX10_mps2_InProc/1  "VINP_CamObsAccX10_mps2_InProc"
  Bus Selector245/1 -> Mux25/1  "<VINP_CamObs1AccXValid_flg_InHAL>"
  Bus Selector246/1 -> Mux25/2  "<VINP_CamObs2AccXValid_flg_InHAL>"
  Bus Selector247/1 -> Mux25/3  "<VINP_CamObs3AccXValid_flg_InHAL>"
  Bus Selector248/1 -> Mux25/4  "<VINP_CamObs4AccXValid_flg_InHAL>"
  Bus Selector249/1 -> Mux25/5  "<VINP_CamObs5AccXValid_flg_InHAL>"
  Bus Selector250/1 -> Mux25/6  "<VINP_CamObs6AccXValid_flg_InHAL>"
  Bus Selector251/1 -> Mux25/7  "<VINP_CamObs7AccXValid_flg_InHAL>"
  Bus Selector252/1 -> Mux25/8  "<VINP_CamObs8AccXValid_flg_InHAL>"
  Bus Selector253/1 -> Mux25/9  "<VINP_CamObs9AccXValid_flg_InHAL>"
  [VINP_Obstacle1_BUS_InHAL] -> Bus Selector245/1
  [VINP_Obstacle2_BUS_InHAL] -> Bus Selector246/1
  [VINP_Obstacle3_BUS_InHAL] -> Bus Selector247/1
  [VINP_Obstacle4_BUS_InHAL] -> Bus Selector248/1
  [VINP_Obstacle5_BUS_InHAL] -> Bus Selector249/1
  [VINP_Obstacle6_BUS_InHAL] -> Bus Selector250/1
  [VINP_Obstacle7_BUS_InHAL] -> Bus Selector251/1
  [VINP_Obstacle8_BUS_InHAL] -> Bus Selector252/1
  [VINP_Obstacle9_BUS_InHAL] -> Bus Selector253/1
  Mux25/1 -> VINP_CamObsAccXValid10_flg_InProc/1  "VINP_CamObsAccXValid10_flg_InProc"
  [VINP_Obstacle10_BUS_InHAL] -> Bus Selector254/1
  Bus Selector254/1 -> Mux25/10  "<VINP_CamObs10AccXValid_flg_InHAL>"
  Bus Selector256/1 -> Mux26/1  "<VINP_CamObs1Ang_deg_InHAL>"
  Bus Selector257/1 -> Mux26/2  "<VINP_CamObs2Ang_deg_InHAL>"
  Bus Selector258/1 -> Mux26/3  "<VINP_CamObs3Ang_deg_InHAL>"
  Bus Selector259/1 -> Mux26/4  "<VINP_CamObs4Ang_deg_InHAL>"
  Bus Selector260/1 -> Mux26/5  "<VINP_CamObs5Ang_deg_InHAL>"
  Bus Selector261/1 -> Mux26/6  "<VINP_CamObs6Ang_deg_InHAL>"
  Bus Selector262/1 -> Mux26/7  "<VINP_CamObs7Ang_deg_InHAL>"
  Bus Selector263/1 -> Mux26/8  "<VINP_CamObs8Ang_deg_InHAL>"
  Bus Selector264/1 -> Mux26/9  "<VINP_CamObs9Ang_deg_InHAL>"
  [VINP_Obstacle1_BUS_InHAL] -> Bus Selector256/1
  [VINP_Obstacle2_BUS_InHAL] -> Bus Selector257/1
  [VINP_Obstacle3_BUS_InHAL] -> Bus Selector258/1
  [VINP_Obstacle4_BUS_InHAL] -> Bus Selector259/1
  [VINP_Obstacle5_BUS_InHAL] -> Bus Selector260/1
  [VINP_Obstacle6_BUS_InHAL] -> Bus Selector261/1
  [VINP_Obstacle7_BUS_InHAL] -> Bus Selector262/1
  [VINP_Obstacle8_BUS_InHAL] -> Bus Selector263/1
  [VINP_Obstacle9_BUS_InHAL] -> Bus Selector264/1
  Mux26/1 -> VINP_CamObsAng10_deg_InProc/1  "VINP_CamObsAng10_deg_InProc"
  [VINP_Obstacle10_BUS_InHAL] -> Bus Selector265/1
  Bus Selector265/1 -> Mux26/10  "<VINP_CamObs10Ang_deg_InHAL>"
  Bus Selector267/1 -> Mux27/1  "<VINP_CamObs1AngValid_flg_InHAL>"
  Bus Selector268/1 -> Mux27/2  "<VINP_CamObs2AngValid_flg_InHAL>"
  Bus Selector269/1 -> Mux27/3  "<VINP_CamObs3AngValid_flg_InHAL>"
  Bus Selector270/1 -> Mux27/4  "<VINP_CamObs4AngValid_flg_InHAL>"
  Bus Selector271/1 -> Mux27/5  "<VINP_CamObs5AngValid_flg_InHAL>"
  Bus Selector272/1 -> Mux27/6  "<VINP_CamObs6AngValid_flg_InHAL>"
  Bus Selector273/1 -> Mux27/7  "<VINP_CamObs7AngValid_flg_InHAL>"
  Bus Selector274/1 -> Mux27/8  "<VINP_CamObs8AngValid_flg_InHAL>"
  Bus Selector275/1 -> Mux27/9  "<VINP_CamObs9AngValid_flg_InHAL>"
  [VINP_Obstacle1_BUS_InHAL] -> Bus Selector267/1
  [VINP_Obstacle2_BUS_InHAL] -> Bus Selector268/1
  [VINP_Obstacle3_BUS_InHAL] -> Bus Selector269/1
  [VINP_Obstacle4_BUS_InHAL] -> Bus Selector270/1
  [VINP_Obstacle5_BUS_InHAL] -> Bus Selector271/1
  [VINP_Obstacle6_BUS_InHAL] -> Bus Selector272/1
  [VINP_Obstacle7_BUS_InHAL] -> Bus Selector273/1
  [VINP_Obstacle8_BUS_InHAL] -> Bus Selector274/1
  [VINP_Obstacle9_BUS_InHAL] -> Bus Selector275/1
  Mux27/1 -> VINP_CamObsAngValid10_flg_InProc/1  "VINP_CamObsAngValid10_flg_InProc"
  [VINP_Obstacle10_BUS_InHAL] -> Bus Selector276/1
  Bus Selector276/1 -> Mux27/10  "<VINP_CamObs10AngValid_flg_InHAL>"
  Bus Selector278/1 -> Mux28/1  "<VINP_CamObs1AngRate_degps_InHAL>"
  Bus Selector279/1 -> Mux28/2  "<VINP_CamObs2AngRate_degps_InHAL>"
  Bus Selector280/1 -> Mux28/3  "<VINP_CamObs3AngRate_degps_InHAL>"
  Bus Selector281/1 -> Mux28/4  "<VINP_CamObs4AngRate_degps_InHAL>"
  Bus Selector282/1 -> Mux28/5  "<VINP_CamObs5AngRate_degps_InHAL>"
  Bus Selector283/1 -> Mux28/6  "<VINP_CamObs6AngRate_degps_InHAL>"
  Bus Selector284/1 -> Mux28/7  "<VINP_CamObs7AngRate_degps_InHAL>"
  Bus Selector285/1 -> Mux28/8  "<VINP_CamObs8AngRate_degps_InHAL>"
  Bus Selector286/1 -> Mux28/9  "<VINP_CamObs9AngRate_degps_InHAL>"
  [VINP_Obstacle1_BUS_InHAL] -> Bus Selector278/1
  [VINP_Obstacle2_BUS_InHAL] -> Bus Selector279/1
  [VINP_Obstacle3_BUS_InHAL] -> Bus Selector280/1
  [VINP_Obstacle4_BUS_InHAL] -> Bus Selector281/1
  [VINP_Obstacle5_BUS_InHAL] -> Bus Selector282/1
  [VINP_Obstacle6_BUS_InHAL] -> Bus Selector283/1
  [VINP_Obstacle7_BUS_InHAL] -> Bus Selector284/1
  [VINP_Obstacle8_BUS_InHAL] -> Bus Selector285/1
  [VINP_Obstacle9_BUS_InHAL] -> Bus Selector286/1
  Mux28/1 -> VINP_CamObsAngRate10_degps_InProc/1  "VINP_CamObsAngRate10_degps_InProc"
  [VINP_Obstacle10_BUS_InHAL] -> Bus Selector287/1
  Bus Selector287/1 -> Mux28/10  "<VINP_CamObs10AngRate_degps_InHAL>"
  Bus Selector289/1 -> Mux29/1  "<VINP_CamObs1AngRateValid_flg_InHAL>"
  Bus Selector290/1 -> Mux29/2  "<VINP_CamObs2AngRateValid_flg_InHAL>"
  Bus Selector291/1 -> Mux29/3  "<VINP_CamObs3AngRateValid_flg_InHAL>"
  Bus Selector292/1 -> Mux29/4  "<VINP_CamObs4AngRateValid_flg_InHAL>"
  Bus Selector293/1 -> Mux29/5  "<VINP_CamObs5AngRateValid_flg_InHAL>"
  Bus Selector294/1 -> Mux29/6  "<VINP_CamObs6AngRateValid_flg_InHAL>"
  Bus Selector295/1 -> Mux29/7  "<VINP_CamObs7AngRateValid_flg_InHAL>"
  Bus Selector296/1 -> Mux29/8  "<VINP_CamObs8AngRateValid_flg_InHAL>"
  Bus Selector297/1 -> Mux29/9  "<VINP_CamObs9AngRateValid_flg_InHAL>"
  [VINP_Obstacle1_BUS_InHAL] -> Bus Selector289/1
  [VINP_Obstacle2_BUS_InHAL] -> Bus Selector290/1
  [VINP_Obstacle3_BUS_InHAL] -> Bus Selector291/1
  [VINP_Obstacle4_BUS_InHAL] -> Bus Selector292/1
  [VINP_Obstacle5_BUS_InHAL] -> Bus Selector293/1
  [VINP_Obstacle6_BUS_InHAL] -> Bus Selector294/1
  [VINP_Obstacle7_BUS_InHAL] -> Bus Selector295/1
  [VINP_Obstacle8_BUS_InHAL] -> Bus Selector296/1
  [VINP_Obstacle9_BUS_InHAL] -> Bus Selector297/1
  Mux29/1 -> VINP_CamObsAngRateValid10_flg_InProc/1  "VINP_CamObsAngRateValid10_flg_InProc"
  [VINP_Obstacle10_BUS_InHAL] -> Bus Selector298/1
  Bus Selector298/1 -> Mux29/10  "<VINP_CamObs10AngRateValid_flg_InHAL>"
  Bus Selector300/1 -> Mux30/1  "<VINP_CamObs1Replaced_flg_InHAL>"
  Bus Selector301/1 -> Mux30/2  "<VINP_CamObs2Replaced_flg_InHAL>"
  Bus Selector302/1 -> Mux30/3  "<VINP_CamObs3Replaced_flg_InHAL>"
  Bus Selector303/1 -> Mux30/4  "<VINP_CamObs4Replaced_flg_InHAL>"
  Bus Selector304/1 -> Mux30/5  "<VINP_CamObs5Replaced_flg_InHAL>"
  Bus Selector305/1 -> Mux30/6  "<VINP_CamObs6Replaced_flg_InHAL>"
  Bus Selector306/1 -> Mux30/7  "<VINP_CamObs7Replaced_flg_InHAL>"
  Bus Selector307/1 -> Mux30/8  "<VINP_CamObs8Replaced_flg_InHAL>"
  Bus Selector308/1 -> Mux30/9  "<VINP_CamObs9Replaced_flg_InHAL>"
  [VINP_Obstacle1_BUS_InHAL] -> Bus Selector300/1
  [VINP_Obstacle2_BUS_InHAL] -> Bus Selector301/1
  [VINP_Obstacle3_BUS_InHAL] -> Bus Selector302/1
  [VINP_Obstacle4_BUS_InHAL] -> Bus Selector303/1
  [VINP_Obstacle5_BUS_InHAL] -> Bus Selector304/1
  [VINP_Obstacle6_BUS_InHAL] -> Bus Selector305/1
  [VINP_Obstacle7_BUS_InHAL] -> Bus Selector306/1
  [VINP_Obstacle8_BUS_InHAL] -> Bus Selector307/1
  [VINP_Obstacle9_BUS_InHAL] -> Bus Selector308/1
  Mux30/1 -> VINP_CamObsReplaced10_flg_InProc/1  "VINP_CamObsReplaced10_flg_InProc"
  [VINP_Obstacle10_BUS_InHAL] -> Bus Selector309/1
  Bus Selector309/1 -> Mux30/10  "<VINP_CamObs10Replaced_flg_InHAL>"
  Bus Selector390/1 -> Mux39/1  "<VINP_CamObs1ReplacedValid_flg_InHAL>"
  Bus Selector391/1 -> Mux39/2  "<VINP_CamObs2ReplacedValid_flg_InHAL>"
  Bus Selector392/1 -> Mux39/3  "<VINP_CamObs3ReplacedValid_flg_InHAL>"
  Bus Selector393/1 -> Mux39/4  "<VINP_CamObs4ReplacedValid_flg_InHAL>"
  Bus Selector394/1 -> Mux39/5  "<VINP_CamObs5ReplacedValid_flg_InHAL>"
  Bus Selector395/1 -> Mux39/6  "<VINP_CamObs6ReplacedValid_flg_InHAL>"
  Bus Selector396/1 -> Mux39/7  "<VINP_CamObs7ReplacedValid_flg_InHAL>"
  Bus Selector397/1 -> Mux39/8  "<VINP_CamObs8ReplacedValid_flg_InHAL>"
  Bus Selector398/1 -> Mux39/9  "<VINP_CamObs9ReplacedValid_flg_InHAL>"
  [VINP_Obstacle1_BUS_InHAL] -> Bus Selector390/1
  [VINP_Obstacle2_BUS_InHAL] -> Bus Selector391/1
  [VINP_Obstacle3_BUS_InHAL] -> Bus Selector392/1
  [VINP_Obstacle4_BUS_InHAL] -> Bus Selector393/1
  [VINP_Obstacle5_BUS_InHAL] -> Bus Selector394/1
  [VINP_Obstacle6_BUS_InHAL] -> Bus Selector395/1
  [VINP_Obstacle7_BUS_InHAL] -> Bus Selector396/1
  [VINP_Obstacle8_BUS_InHAL] -> Bus Selector397/1
  [VINP_Obstacle9_BUS_InHAL] -> Bus Selector398/1
  Mux39/1 -> VINP_CamObsReplacedValid10_flg_InProc/1  "VINP_CamObsReplacedValid10_flg_InProc"
  [VINP_Obstacle10_BUS_InHAL] -> Bus Selector399/1
  Bus Selector399/1 -> Mux39/10  "<VINP_CamObs10ReplacedValid_flg_InHAL>"
